simulink model "BswSigProc"  (R2022b) (continued)
== system BswSigProc == (continued)
  [SysServCarCfgSts_SysServDUIncarMotorSts_Tag] -> BswSigProc_Runnable_sys/93(SysServCarCfgSts_SysServDUIncarMotorSts_read)
  [SysServCarCfgSts_SysServDUIncarMotorSts_Tag] -> BswSigProc_SignalDeal_sys/48(SysServCarCfgSts_SysServDUIncarMotorSts_read)
  SysServCarCfgSts_SysServDUFRVentSts/1 -> [SysServCarCfgSts_SysServDUFRVentSts_Tag]
  [SysServCarCfgSts_SysServDUFRVentSts_Tag] -> BswSigProc_Runnable_sys/92(SysServCarCfgSts_SysServDUFRVentSts_read)
  [SysServCarCfgSts_SysServDUFRVentSts_Tag] -> BswSigProc_SignalDeal_sys/47(SysServCarCfgSts_SysServDUFRVentSts_read)
  SysServCarCfgSts_SysServDUFRFootSts/1 -> [SysServCarCfgSts_SysServDUFRFootSts_Tag]
  [SysServCarCfgSts_SysServDUFRFootSts_Tag] -> BswSigProc_Runnable_sys/91(SysServCarCfgSts_SysServDUFRFootSts_read)
  [SysServCarCfgSts_SysServDUFRFootSts_Tag] -> BswSigProc_SignalDeal_sys/46(SysServCarCfgSts_SysServDUFRFootSts_read)
  SysServCarCfgSts_SysServDUFLVentSts/1 -> [SysServCarCfgSts_SysServDUFLVentSts_Tag]
  [SysServCarCfgSts_SysServDUFLVentSts_Tag] -> BswSigProc_Runnable_sys/90(SysServCarCfgSts_SysServDUFLVentSts_read)
  [SysServCarCfgSts_SysServDUFLVentSts_Tag] -> BswSigProc_SignalDeal_sys/45(SysServCarCfgSts_SysServDUFLVentSts_read)
  SysServCarCfgSts_SysServDUFLFootSts/1 -> [SysServCarCfgSts_SysServDUFLFootSts_Tag]
  [SysServCarCfgSts_SysServDUFLFootSts_Tag] -> BswSigProc_Runnable_sys/89(SysServCarCfgSts_SysServDUFLFootSts_read)
  [SysServCarCfgSts_SysServDUFLFootSts_Tag] -> BswSigProc_SignalDeal_sys/44(SysServCarCfgSts_SysServDUFLFootSts_read)
  SysServCarCfgSts_SysServDUDCTRSts/1 -> [SysServCarCfgSts_SysServDUDCTRSts_Tag]
  [SysServCarCfgSts_SysServDUDCTRSts_Tag] -> BswSigProc_Runnable_sys/88(SysServCarCfgSts_SysServDUDCTRSts_read)
  [SysServCarCfgSts_SysServDUDCTRSts_Tag] -> BswSigProc_SignalDeal_sys/43(SysServCarCfgSts_SysServDUDCTRSts_read)
  SysServCarCfgSts_SysServDUDCTLSts/1 -> [SysServCarCfgSts_SysServDUDCTLSts_Tag]
  [SysServCarCfgSts_SysServDUDCTLSts_Tag] -> BswSigProc_Runnable_sys/87(SysServCarCfgSts_SysServDUDCTLSts_read)
  [SysServCarCfgSts_SysServDUDCTLSts_Tag] -> BswSigProc_SignalDeal_sys/42(SysServCarCfgSts_SysServDUDCTLSts_read)
  SysServCarCfgSts_SysServDUDCSTLSts/1 -> [SysServCarCfgSts_SysServDUDCSTLSts_Tag]
  [SysServCarCfgSts_SysServDUDCSTLSts_Tag] -> BswSigProc_Runnable_sys/86(SysServCarCfgSts_SysServDUDCSTLSts_read)
  [SysServCarCfgSts_SysServDUDCSTLSts_Tag] -> BswSigProc_SignalDeal_sys/41(SysServCarCfgSts_SysServDUDCSTLSts_read)
  SysServCarCfgSts_SysServDUDCSModeSts/1 -> [SysServCarCfgSts_SysServDUDCSModeSts_Tag]
  [SysServCarCfgSts_SysServDUDCSModeSts_Tag] -> BswSigProc_Runnable_sys/85(SysServCarCfgSts_SysServDUDCSModeSts_read)
  [SysServCarCfgSts_SysServDUDCSModeSts_Tag] -> BswSigProc_SignalDeal_sys/40(SysServCarCfgSts_SysServDUDCSModeSts_read)
  SysServCarCfgSts_SysServDUDCRecSts/1 -> [SysServCarCfgSts_SysServDUDCRecSts_Tag]
  [SysServCarCfgSts_SysServDUDCRecSts_Tag] -> BswSigProc_Runnable_sys/84(SysServCarCfgSts_SysServDUDCRecSts_read)
  [SysServCarCfgSts_SysServDUDCRecSts_Tag] -> BswSigProc_SignalDeal_sys/39(SysServCarCfgSts_SysServDUDCRecSts_read)
  SysServCarCfgSts_SysServDUDCModeSts/1 -> [SysServCarCfgSts_SysServDUDCModeSts_Tag]
  [SysServCarCfgSts_SysServDUDCModeSts_Tag] -> BswSigProc_Runnable_sys/83(SysServCarCfgSts_SysServDUDCModeSts_read)
  [SysServCarCfgSts_SysServDUDCModeSts_Tag] -> BswSigProc_SignalDeal_sys/38(SysServCarCfgSts_SysServDUDCModeSts_read)
  SysServCarCfgSts_SysServDUDCFLMdeSts/1 -> [SysServCarCfgSts_SysServDUDCFLMdeSts_Tag]
  [SysServCarCfgSts_SysServDUDCFLMdeSts_Tag] -> BswSigProc_Runnable_sys/82(SysServCarCfgSts_SysServDUDCFLMdeSts_read)
  [SysServCarCfgSts_SysServDUDCFLMdeSts_Tag] -> BswSigProc_SignalDeal_sys/37(SysServCarCfgSts_SysServDUDCFLMdeSts_read)
  SysServCarCfgSts_SysServDUDCDefrostSts/1 -> [SysServCarCfgSts_SysServDUDCDefrostSts_Tag]
  [SysServCarCfgSts_SysServDUDCDefrostSts_Tag] -> BswSigProc_Runnable_sys/81(SysServCarCfgSts_SysServDUDCDefrostSts_read)
  [SysServCarCfgSts_SysServDUDCDefrostSts_Tag] -> BswSigProc_SignalDeal_sys/36(SysServCarCfgSts_SysServDUDCDefrostSts_read)
  SysServCarCfgSts_SysServDUBlowerPwmSts/1 -> [SysServCarCfgSts_SysServDUBlowerPwmSts_Tag]
  [SysServCarCfgSts_SysServDUBlowerPwmSts_Tag] -> BswSigProc_Runnable_sys/80(SysServCarCfgSts_SysServDUBlowerPwmSts_read)
  [SysServCarCfgSts_SysServDUBlowerPwmSts_Tag] -> BswSigProc_SignalDeal_sys/35(SysServCarCfgSts_SysServDUBlowerPwmSts_read)
  SysServCarCfgSts_SysServDUBlowerPwmSignSts/1 -> [SysServCarCfgSts_SysServDUBlowerPwmSignSts_Tag]
  [SysServCarCfgSts_SysServDUBlowerPwmSignSts_Tag] -> BswSigProc_Runnable_sys/78(SysServCarCfgSts_SysServDUBlowerPwmSignSts_read)
  [SysServCarCfgSts_SysServDUBlowerPwmSignSts_Tag] -> BswSigProc_SignalDeal_sys/34(SysServCarCfgSts_SysServDUBlowerPwmSignSts_read)
  SysServCarCfgSts_SysServDUAirVentSnrSts/1 -> [SysServCarCfgSts_SysServDUAirVentSnrSts_Tag]
  [SysServCarCfgSts_SysServDUAirVentSnrSts_Tag] -> BswSigProc_Runnable_sys/77(SysServCarCfgSts_SysServDUAirVentSnrSts_read)
  [SysServCarCfgSts_SysServDUAirVentSnrSts_Tag] -> BswSigProc_SignalDeal_sys/33(SysServCarCfgSts_SysServDUAirVentSnrSts_read)
  SysServCarCfgSts_SysServCCPLin3VGASts/1 -> [SysServCarCfgSts_SysServCCPLin3VGASts_Tag]
  [SysServCarCfgSts_SysServCCPLin3VGASts_Tag] -> BswSigProc_Runnable_sys/76(SysServCarCfgSts_SysServCCPLin3VGASts_read)
  [SysServCarCfgSts_SysServCCPLin3VGASts_Tag] -> BswSigProc_SignalDeal_sys/32(SysServCarCfgSts_SysServCCPLin3VGASts_read)
  SysServCarCfgSts_SysServCCPLin2PMSISts/1 -> [SysServCarCfgSts_SysServCCPLin2PMSISts_Tag]
  [SysServCarCfgSts_SysServCCPLin2PMSISts_Tag] -> BswSigProc_Runnable_sys/75(SysServCarCfgSts_SysServCCPLin2PMSISts_read)
  [SysServCarCfgSts_SysServCCPLin2PMSISts_Tag] -> BswSigProc_SignalDeal_sys/31(SysServCarCfgSts_SysServCCPLin2PMSISts_read)
  SysServCarCfgSts_SysServCCPLin2CDSSts/1 -> [SysServCarCfgSts_SysServCCPLin2CDSSts_Tag]
  [SysServCarCfgSts_SysServCCPLin2CDSSts_Tag] -> BswSigProc_Runnable_sys/74(SysServCarCfgSts_SysServCCPLin2CDSSts_read)
  [SysServCarCfgSts_SysServCCPLin2CDSSts_Tag] -> BswSigProc_SignalDeal_sys/30(SysServCarCfgSts_SysServCCPLin2CDSSts_read)
  SysServCarCfgSts_SysServCCPLin2AIUSts/1 -> [SysServCarCfgSts_SysServCCPLin2AIUSts_Tag]
  [SysServCarCfgSts_SysServCCPLin2AIUSts_Tag] -> BswSigProc_Runnable_sys/72(SysServCarCfgSts_SysServCCPLin2AIUSts_read)
  [SysServCarCfgSts_SysServCCPLin2AIUSts_Tag] -> BswSigProc_SignalDeal_sys/29(SysServCarCfgSts_SysServCCPLin2AIUSts_read)
  SysServCarCfgSts_SysServCCPLin2AFUSts/1 -> [SysServCarCfgSts_SysServCCPLin2AFUSts_Tag]
  [SysServCarCfgSts_SysServCCPLin2AFUSts_Tag] -> BswSigProc_Runnable_sys/71(SysServCarCfgSts_SysServCCPLin2AFUSts_read)
  [SysServCarCfgSts_SysServCCPLin2AFUSts_Tag] -> BswSigProc_SignalDeal_sys/28(SysServCarCfgSts_SysServCCPLin2AFUSts_read)
  SysServCarCfgSts_SysServCCPHEXSensorSts/1 -> [SysServCarCfgSts_SysServCCPHEXSensorSts_Tag]
  [SysServCarCfgSts_SysServCCPHEXSensorSts_Tag] -> BswSigProc_Runnable_sys/70(SysServCarCfgSts_SysServCCPHEXSensorSts_read)
  [SysServCarCfgSts_SysServCCPHEXSensorSts_Tag] -> BswSigProc_SignalDeal_sys/27(SysServCarCfgSts_SysServCCPHEXSensorSts_read)
  SysServCarCfgSts_SysServCCPBGMNum/1 -> [SysServCarCfgSts_SysServCCPBGMNum_Tag]
  [SysServCarCfgSts_SysServCCPBGMNum_Tag] -> BswSigProc_Runnable_sys/69(SysServCarCfgSts_SysServCCPBGMNum_read)
  [SysServCarCfgSts_SysServCCPBGMNum_Tag] -> BswSigProc_SignalDeal_sys/99(SysServCarCfgSts_SysServCCPBGMNum_read)
  SysServCarCfgSts_SysServCCPAQSSts/1 -> [SysServCarCfgSts_SysServCCPAQSSts_Tag]
  [SysServCarCfgSts_SysServCCPAQSSts_Tag] -> BswSigProc_Runnable_sys/68(SysServCarCfgSts_SysServCCPAQSSts_read)
  [SysServCarCfgSts_SysServCCPAQSSts_Tag] -> BswSigProc_SignalDeal_sys/26(SysServCarCfgSts_SysServCCPAQSSts_read)
  SysServCarCfgSts_SysServCarID/1 -> [SysServCarCfgSts_SysServCarID_Tag]
  [SysServCarCfgSts_SysServCarID_Tag] -> BswSigProc_Runnable_sys/67(SysServCarCfgSts_SysServCarID_read)
  [SysServCarCfgSts_SysServCarID_Tag] -> BswSigProc_SignalDeal_sys/25(SysServCarCfgSts_SysServCarID_read)
  RlyCrashForClimaReq_Ngcc_RlyCrashForClimaReq/1 -> [RlyCrashForClimaReq_Ngcc_RlyCrashForClimaReq_Tag]
  [RlyCrashForClimaReq_Ngcc_RlyCrashForClimaReq_Tag] -> BswSigProc_Runnable_sys/66(RlyCrashForClimaReq_Ngcc_RlyCrashForClimaReq_read)
  [RlyCrashForClimaReq_Ngcc_RlyCrashForClimaReq_Tag] -> BswSigProc_SignalDeal_sys/24(RlyCrashForClimaReq_Ngcc_RlyCrashForClimaReq_read)
  RlyCmftForClimaReq_Ngcc_RlyCmftForClimaReq/1 -> [RlyCmftForClimaReq_Ngcc_RlyCmftForClimaReq_Tag]
  [RlyCmftForClimaReq_Ngcc_RlyCmftForClimaReq_Tag] -> BswSigProc_Runnable_sys/65(RlyCmftForClimaReq_Ngcc_RlyCmftForClimaReq_read)
  [RlyCmftForClimaReq_Ngcc_RlyCmftForClimaReq_Tag] -> BswSigProc_SignalDeal_sys/23(RlyCmftForClimaReq_Ngcc_RlyCmftForClimaReq_read)
  IntCo2RunSts_IntCo2RunSts_IsUpdated/1 -> [IntCo2RunSts_IntCo2RunSts_IsUpdated_Tag]
  [IntCo2RunSts_IntCo2RunSts_IsUpdated_Tag] -> BswSigProc_Runnable_sys/64(IntCo2RunSts_IntCo2RunSts_IsUpdated_read)
  [IntCo2RunSts_IntCo2RunSts_IsUpdated_Tag] -> BswSigProc_SignalDeal_sys/81(IntCo2RunSts_IntCo2RunSts_IsUpdated_read)
  IntCo2RunSts_IntCo2RunSts/1 -> [IntCo2RunSts_IntCo2RunSts_Tag]
  [IntCo2RunSts_IntCo2RunSts_Tag] -> BswSigProc_Runnable_sys/63(IntCo2RunSts_IntCo2RunSts_read)
  [IntCo2RunSts_IntCo2RunSts_Tag] -> BswSigProc_SignalDeal_sys/80(IntCo2RunSts_IntCo2RunSts_read)
  IntCo2Conc_IntCo2Conc_IsUpdated/1 -> [IntCo2Conc_IntCo2Conc_IsUpdated_Tag]
  [IntCo2Conc_IntCo2Conc_IsUpdated_Tag] -> BswSigProc_Runnable_sys/62(IntCo2Conc_IntCo2Conc_IsUpdated_read)
  [IntCo2Conc_IntCo2Conc_IsUpdated_Tag] -> BswSigProc_SignalDeal_sys/79(IntCo2Conc_IntCo2Conc_IsUpdated_read)
  IntCo2Conc_IntCo2Conc/1 -> [IntCo2Conc_IntCo2Conc_Tag]
  [IntCo2Conc_IntCo2Conc_Tag] -> BswSigProc_Runnable_sys/61(IntCo2Conc_IntCo2Conc_read)
  [IntCo2Conc_IntCo2Conc_Tag] -> BswSigProc_SignalDeal_sys/78(IntCo2Conc_IntCo2Conc_read)
  HvacOsaAndRecActrQfForHp_HvacOsaAndRecActrQfForHp/1 -> [HvacOsaAndRecActrQfForHp_HvacOsaAndRecActrQfForHp_Tag]
  [HvacOsaAndRecActrQfForHp_HvacOsaAndRecActrQfForHp_Tag] -> BswSigProc_Runnable_sys/59(HvacOsaAndRecActrQfForHp_HvacOsaAndRecActrQfForHp_read)
  [HvacOsaAndRecActrQfForHp_HvacOsaAndRecActrQfForHp_Tag] -> BswSigProc_SignalDeal_sys/21(HvacOsaAndRecActrQfForHp_HvacOsaAndRecActrQfForHp_read)
  HvacFanSts3HvacFanSpdFd_HvacFanSts3HvacFanSpdFd_IsUpdated/1 -> [HvacFanSts3HvacFanSpdFd_HvacFanSts3HvacFanSpdFd_IsUpdated_Tag]
  [HvacFanSts3HvacFanSpdFd_HvacFanSts3HvacFanSpdFd_IsUpdated_Tag] -> BswSigProc_Runnable_sys/58(HvacFanSts3HvacFanSpdFd_HvacFanSts3HvacFanSpdFd_IsUpdated_read)
  [HvacFanSts3HvacFanSpdFd_HvacFanSts3HvacFanSpdFd_IsUpdated_Tag] -> BswSigProc_SignalDeal_sys/77(HvacFanSts3HvacFanSpdFd_HvacFanSts3HvacFanSpdFd_IsUpdated_read)
  HvacFanSts3HvacFanSpdFd_HvacFanSts3HvacFanSpdFd/1 -> [HvacFanSts3HvacFanSpdFd_HvacFanSts3HvacFanSpdFd_Tag]
  [HvacFanSts3HvacFanSpdFd_HvacFanSts3HvacFanSpdFd_Tag] -> BswSigProc_Runnable_sys/57(HvacFanSts3HvacFanSpdFd_HvacFanSts3HvacFanSpdFd_read)
  [HvacFanSts3HvacFanSpdFd_HvacFanSts3HvacFanSpdFd_Tag] -> BswSigProc_SignalDeal_sys/76(HvacFanSts3HvacFanSpdFd_HvacFanSts3HvacFanSpdFd_read)
  HvacFanSts3HvacFanBattU_HvacFanSts3HvacFanBattU_IsUpdated/1 -> [HvacFanSts3HvacFanBattU_HvacFanSts3HvacFanBattU_IsUpdated_Tag]
  [HvacFanSts3HvacFanBattU_HvacFanSts3HvacFanBattU_IsUpdated_Tag] -> BswSigProc_Runnable_sys/56(HvacFanSts3HvacFanBattU_HvacFanSts3HvacFanBattU_IsUpdated_read)
  [HvacFanSts3HvacFanBattU_HvacFanSts3HvacFanBattU_IsUpdated_Tag] -> BswSigProc_SignalDeal_sys/75(HvacFanSts3HvacFanBattU_HvacFanSts3HvacFanBattU_IsUpdated_read)
  HvacFanSts3HvacFanBattU_HvacFanSts3HvacFanBattU/1 -> [HvacFanSts3HvacFanBattU_HvacFanSts3HvacFanBattU_Tag]
  [HvacFanSts3HvacFanBattU_HvacFanSts3HvacFanBattU_Tag] -> BswSigProc_Runnable_sys/55(HvacFanSts3HvacFanBattU_HvacFanSts3HvacFanBattU_read)
  [HvacFanSts3HvacFanBattU_HvacFanSts3HvacFanBattU_Tag] -> BswSigProc_SignalDeal_sys/74(HvacFanSts3HvacFanBattU_HvacFanSts3HvacFanBattU_read)
  HvacFanSts3HvacFanBattI_HvacFanSts3HvacFanBattI_IsUpdated/1 -> [HvacFanSts3HvacFanBattI_HvacFanSts3HvacFanBattI_IsUpdated_Tag]
  [HvacFanSts3HvacFanBattI_HvacFanSts3HvacFanBattI_IsUpdated_Tag] -> BswSigProc_Runnable_sys/54(HvacFanSts3HvacFanBattI_HvacFanSts3HvacFanBattI_IsUpdated_read)
  [HvacFanSts3HvacFanBattI_HvacFanSts3HvacFanBattI_IsUpdated_Tag] -> BswSigProc_SignalDeal_sys/73(HvacFanSts3HvacFanBattI_HvacFanSts3HvacFanBattI_IsUpdated_read)
  HvacFanSts3HvacFanBattI_HvacFanSts3HvacFanBattI/1 -> [HvacFanSts3HvacFanBattI_HvacFanSts3HvacFanBattI_Tag]
  [HvacFanSts3HvacFanBattI_HvacFanSts3HvacFanBattI_Tag] -> BswSigProc_Runnable_sys/53(HvacFanSts3HvacFanBattI_HvacFanSts3HvacFanBattI_read)
  [HvacFanSts3HvacFanBattI_HvacFanSts3HvacFanBattI_Tag] -> BswSigProc_SignalDeal_sys/72(HvacFanSts3HvacFanBattI_HvacFanSts3HvacFanBattI_read)
  HvacFanSts2HvacFanBattU2_HvacFanSts2HvacFanBattU2_IsUpdated/1 -> [HvacFanSts2HvacFanBattU2_HvacFanSts2HvacFanBattU2_IsUpdated_Tag]
  [HvacFanSts2HvacFanBattU2_HvacFanSts2HvacFanBattU2_IsUpdated_Tag] -> BswSigProc_Runnable_sys/52(HvacFanSts2HvacFanBattU2_HvacFanSts2HvacFanBattU2_IsUpdated_read)
  [HvacFanSts2HvacFanBattU2_HvacFanSts2HvacFanBattU2_IsUpdated_Tag] -> BswSigProc_SignalDeal_sys/71(HvacFanSts2HvacFanBattU2_HvacFanSts2HvacFanBattU2_IsUpdated_read)
  HvacFanSts2HvacFanBattU2_HvacFanSts2HvacFanBattU2/1 -> [HvacFanSts2HvacFanBattU2_HvacFanSts2HvacFanBattU2_Tag]
  [HvacFanSts2HvacFanBattU2_HvacFanSts2HvacFanBattU2_Tag] -> BswSigProc_Runnable_sys/51(HvacFanSts2HvacFanBattU2_HvacFanSts2HvacFanBattU2_read)
  [HvacFanSts2HvacFanBattU2_HvacFanSts2HvacFanBattU2_Tag] -> BswSigProc_SignalDeal_sys/70(HvacFanSts2HvacFanBattU2_HvacFanSts2HvacFanBattU2_read)
  HvacFanSts2HvacFanBattI2_HvacFanSts2HvacFanBattI2_IsUpdated/1 -> [HvacFanSts2HvacFanBattI2_HvacFanSts2HvacFanBattI2_IsUpdated_Tag]
  [HvacFanSts2HvacFanBattI2_HvacFanSts2HvacFanBattI2_IsUpdated_Tag] -> BswSigProc_Runnable_sys/50(HvacFanSts2HvacFanBattI2_HvacFanSts2HvacFanBattI2_IsUpdated_read)
  [HvacFanSts2HvacFanBattI2_HvacFanSts2HvacFanBattI2_IsUpdated_Tag] -> BswSigProc_SignalDeal_sys/69(HvacFanSts2HvacFanBattI2_HvacFanSts2HvacFanBattI2_IsUpdated_read)
  HvacFanSts2HvacFanBattI2_HvacFanSts2HvacFanBattI2/1 -> [HvacFanSts2HvacFanBattI2_HvacFanSts2HvacFanBattI2_Tag]
  [HvacFanSts2HvacFanBattI2_HvacFanSts2HvacFanBattI2_Tag] -> BswSigProc_Runnable_sys/49(HvacFanSts2HvacFanBattI2_HvacFanSts2HvacFanBattI2_read)
  [HvacFanSts2HvacFanBattI2_HvacFanSts2HvacFanBattI2_Tag] -> BswSigProc_SignalDeal_sys/68(HvacFanSts2HvacFanBattI2_HvacFanSts2HvacFanBattI2_read)
  HmiHvacFanLvlFrnt_HmiHvacFanLvlFrnt/1 -> [HmiHvacFanLvlFrnt_HmiHvacFanLvlFrnt_Tag]
  [HmiHvacFanLvlFrnt_HmiHvacFanLvlFrnt_Tag] -> BswSigProc_Runnable_sys/48(HmiHvacFanLvlFrnt_HmiHvacFanLvlFrnt_read)
  [HmiHvacFanLvlFrnt_HmiHvacFanLvlFrnt_Tag] -> BswSigProc_SignalDeal_sys/92(HmiHvacFanLvlFrnt_HmiHvacFanLvlFrnt_read)
  FrntHvacBlowerSts_FrntHvacBlowerSts/1 -> [FrntHvacBlowerSts_FrntHvacBlowerSts_Tag]
  [FrntHvacBlowerSts_FrntHvacBlowerSts_Tag] -> BswSigProc_Runnable_sys/47(FrntHvacBlowerSts_FrntHvacBlowerSts_read)
  [FrntHvacBlowerSts_FrntHvacBlowerSts_Tag] -> BswSigProc_SignalDeal_sys/20(FrntHvacBlowerSts_FrntHvacBlowerSts_read)
  CmptmtTRearRaw_CmptmtTRearRaw/1 -> [CmptmtTRearRaw_CmptmtTRearRaw_Tag]
  [CmptmtTRearRaw_CmptmtTRearRaw_Tag] -> BswSigProc_Runnable_sys/45(CmptmtTRearRaw_CmptmtTRearRaw_read)
  [CmptmtTRearRaw_CmptmtTRearRaw_Tag] -> BswSigProc_SignalDeal_sys/91(CmptmtTRearRaw_CmptmtTRearRaw_read)
  CarTiGlb_CarTiGlb_IsUpdated/1 -> [CarTiGlb_CarTiGlb_IsUpdated_Tag]
  [CarTiGlb_CarTiGlb_IsUpdated_Tag] -> BswSigProc_Runnable_sys/44(CarTiGlb_CarTiGlb_IsUpdated_read)
  [CarTiGlb_CarTiGlb_IsUpdated_Tag] -> BswSigProc_SignalDeal_sys/88(CarTiGlb_CarTiGlb_IsUpdated_read)
  CarTiGlb_CarTiGlb/1 -> [CarTiGlb_CarTiGlb_Tag]
  [CarTiGlb_CarTiGlb_Tag] -> BswSigProc_Runnable_sys/43(CarTiGlb_CarTiGlb_read)
  [CarTiGlb_CarTiGlb_Tag] -> BswSigProc_SignalDeal_sys/87(CarTiGlb_CarTiGlb_read)
  BkpOfDstTrvld_BkpOfDstTrvld_IsUpdated/1 -> [BkpOfDstTrvld_BkpOfDstTrvld_IsUpdated_Tag]
  [BkpOfDstTrvld_BkpOfDstTrvld_IsUpdated_Tag] -> BswSigProc_Runnable_sys/42(BkpOfDstTrvld_BkpOfDstTrvld_IsUpdated_read)
  [BkpOfDstTrvld_BkpOfDstTrvld_IsUpdated_Tag] -> BswSigProc_SignalDeal_sys/86(BkpOfDstTrvld_BkpOfDstTrvld_IsUpdated_read)
  BkpOfDstTrvld_BkpOfDstTrvld/1 -> [BkpOfDstTrvld_BkpOfDstTrvld_Tag]
  [BkpOfDstTrvld_BkpOfDstTrvld_Tag] -> BswSigProc_Runnable_sys/41(BkpOfDstTrvld_BkpOfDstTrvld_read)
  [BkpOfDstTrvld_BkpOfDstTrvld_Tag] -> BswSigProc_SignalDeal_sys/85(BkpOfDstTrvld_BkpOfDstTrvld_read)
  BatteryVolValue_BatteryVolValue/1 -> [BatteryVolValue_BatteryVolValue_Tag]
  [BatteryVolValue_BatteryVolValue_Tag] -> BswSigProc_Runnable_sys/40(BatteryVolValue_BatteryVolValue_read)
  [BatteryVolValue_BatteryVolValue_Tag] -> BswSigProc_SignalDeal_sys/18(BatteryVolValue_BatteryVolValue_read)
  AmbTRaw_AmbTRaw/1 -> [AmbTRaw_AmbTRaw_Tag]
  [AmbTRaw_AmbTRaw_Tag] -> BswSigProc_Runnable_sys/39(AmbTRaw_AmbTRaw_read)
  [AmbTRaw_AmbTRaw_Tag] -> BswSigProc_SignalDeal_sys/84(AmbTRaw_AmbTRaw_read)
  AirFragCh5RunngSts_AirFragCh5RunngSts_IsUpdated/1 -> [AirFragCh5RunngSts_AirFragCh5RunngSts_IsUpdated_Tag]
  [AirFragCh5RunngSts_AirFragCh5RunngSts_IsUpdated_Tag] -> BswSigProc_Runnable_sys/38(AirFragCh5RunngSts_AirFragCh5RunngSts_IsUpdated_read)
  [AirFragCh5RunngSts_AirFragCh5RunngSts_IsUpdated_Tag] -> BswSigProc_SignalDeal_sys/67(AirFragCh5RunngSts_AirFragCh5RunngSts_IsUpdated_read)
  AirFragCh5RunngSts_AirFragCh5RunngSts/1 -> [AirFragCh5RunngSts_AirFragCh5RunngSts_Tag]
  [AirFragCh5RunngSts_AirFragCh5RunngSts_Tag] -> BswSigProc_Runnable_sys/37(AirFragCh5RunngSts_AirFragCh5RunngSts_read)
  [AirFragCh5RunngSts_AirFragCh5RunngSts_Tag] -> BswSigProc_SignalDeal_sys/66(AirFragCh5RunngSts_AirFragCh5RunngSts_read)
  AirFragCh4RunngSts_AirFragCh4RunngSts_IsUpdated/1 -> [AirFragCh4RunngSts_AirFragCh4RunngSts_IsUpdated_Tag]
  [AirFragCh4RunngSts_AirFragCh4RunngSts_IsUpdated_Tag] -> BswSigProc_Runnable_sys/36(AirFragCh4RunngSts_AirFragCh4RunngSts_IsUpdated_read)
  [AirFragCh4RunngSts_AirFragCh4RunngSts_IsUpdated_Tag] -> BswSigProc_SignalDeal_sys/65(AirFragCh4RunngSts_AirFragCh4RunngSts_IsUpdated_read)
  AirFragCh4RunngSts_AirFragCh4RunngSts/1 -> [AirFragCh4RunngSts_AirFragCh4RunngSts_Tag]
  [AirFragCh4RunngSts_AirFragCh4RunngSts_Tag] -> BswSigProc_Runnable_sys/35(AirFragCh4RunngSts_AirFragCh4RunngSts_read)
  [AirFragCh4RunngSts_AirFragCh4RunngSts_Tag] -> BswSigProc_SignalDeal_sys/64(AirFragCh4RunngSts_AirFragCh4RunngSts_read)
  AirFragCh3RunngSts_AirFragCh3RunngSts_IsUpdated/1 -> [AirFragCh3RunngSts_AirFragCh3RunngSts_IsUpdated_Tag]
  [AirFragCh3RunngSts_AirFragCh3RunngSts_IsUpdated_Tag] -> BswSigProc_Runnable_sys/34(AirFragCh3RunngSts_AirFragCh3RunngSts_IsUpdated_read)
  [AirFragCh3RunngSts_AirFragCh3RunngSts_IsUpdated_Tag] -> BswSigProc_SignalDeal_sys/63(AirFragCh3RunngSts_AirFragCh3RunngSts_IsUpdated_read)
  AirFragCh3RunngSts_AirFragCh3RunngSts/1 -> [AirFragCh3RunngSts_AirFragCh3RunngSts_Tag]
  [AirFragCh3RunngSts_AirFragCh3RunngSts_Tag] -> BswSigProc_Runnable_sys/33(AirFragCh3RunngSts_AirFragCh3RunngSts_read)
  [AirFragCh3RunngSts_AirFragCh3RunngSts_Tag] -> BswSigProc_SignalDeal_sys/62(AirFragCh3RunngSts_AirFragCh3RunngSts_read)
  AirFragCh2RunngSts_AirFragCh2RunngSts_IsUpdated/1 -> [AirFragCh2RunngSts_AirFragCh2RunngSts_IsUpdated_Tag]
  [AirFragCh2RunngSts_AirFragCh2RunngSts_IsUpdated_Tag] -> BswSigProc_Runnable_sys/32(AirFragCh2RunngSts_AirFragCh2RunngSts_IsUpdated_read)
  [AirFragCh2RunngSts_AirFragCh2RunngSts_IsUpdated_Tag] -> BswSigProc_SignalDeal_sys/61(AirFragCh2RunngSts_AirFragCh2RunngSts_IsUpdated_read)
  AirFragCh2RunngSts_AirFragCh2RunngSts/1 -> [AirFragCh2RunngSts_AirFragCh2RunngSts_Tag]
  [AirFragCh2RunngSts_AirFragCh2RunngSts_Tag] -> BswSigProc_Runnable_sys/31(AirFragCh2RunngSts_AirFragCh2RunngSts_read)
  [AirFragCh2RunngSts_AirFragCh2RunngSts_Tag] -> BswSigProc_SignalDeal_sys/60(AirFragCh2RunngSts_AirFragCh2RunngSts_read)
  AirFragCh1RunngSts_AirFragCh1RunngSts_IsUpdated/1 -> [AirFragCh1RunngSts_AirFragCh1RunngSts_IsUpdated_Tag]
  [AirFragCh1RunngSts_AirFragCh1RunngSts_IsUpdated_Tag] -> BswSigProc_Runnable_sys/30(AirFragCh1RunngSts_AirFragCh1RunngSts_IsUpdated_read)
  [AirFragCh1RunngSts_AirFragCh1RunngSts_IsUpdated_Tag] -> BswSigProc_SignalDeal_sys/83(AirFragCh1RunngSts_AirFragCh1RunngSts_IsUpdated_read)
  AirFragCh1RunngSts_AirFragCh1RunngSts/1 -> [AirFragCh1RunngSts_AirFragCh1RunngSts_Tag]
  [AirFragCh1RunngSts_AirFragCh1RunngSts_Tag] -> BswSigProc_Runnable_sys/29(AirFragCh1RunngSts_AirFragCh1RunngSts_read)
  [AirFragCh1RunngSts_AirFragCh1RunngSts_Tag] -> BswSigProc_SignalDeal_sys/82(AirFragCh1RunngSts_AirFragCh1RunngSts_read)
  AirFlapPosnFrntActForRowSecForActrTRi_AirFlapPosnFrntActForRowSecForActrTRi/1 -> [AirFlapPosnFrntActForRowSecForActrTRi_AirFlapP_9fab42d88a26ba35]
  [AirFlapPosnFrntActForRowSecForActrTRi_AirFlapP_9fab42d88a26ba35] -> BswSigProc_Runnable_sys/28(AirFlapPosnFrntActForRowSecForActrTRi_AirFlapPosnFrntActForRowSecForActrTRi_read)
  [AirFlapPosnFrntActForRowSecForActrTRi_AirFlapP_9fab42d88a26ba35] -> BswSigProc_SignalDeal_sys/17(AirFlapPosnFrntActForRowSecForActrTRi_AirFlapPosnFrntActForRowSecForActrTRi_read)
  AirFlapPosnFrntActForRowSecForActrTLe_AirFlapPosnFrntActForRowSecForActrTLe/1 -> [AirFlapPosnFrntActForRowSecForActrTLe_AirFlapP_d65546ff01a636a1]
  [AirFlapPosnFrntActForRowSecForActrTLe_AirFlapP_d65546ff01a636a1] -> BswSigProc_Runnable_sys/27(AirFlapPosnFrntActForRowSecForActrTLe_AirFlapPosnFrntActForRowSecForActrTLe_read)
  [AirFlapPosnFrntActForRowSecForActrTLe_AirFlapP_d65546ff01a636a1] -> BswSigProc_SignalDeal_sys/16(AirFlapPosnFrntActForRowSecForActrTLe_AirFlapPosnFrntActForRowSecForActrTLe_read)
  AirFlapPosnFrntActForRowFirstForRecirc_AirFlapPosnFrntActForRowFirstForRecirc/1 -> [AirFlapPosnFrntActForRowFirstForRecirc_AirFlap_171d170c661908da]
  [AirFlapPosnFrntActForRowFirstForRecirc_AirFlap_171d170c661908da] -> BswSigProc_Runnable_sys/26(AirFlapPosnFrntActForRowFirstForRecirc_AirFlapPosnFrntActForRowFirstForRecirc_read)
  [AirFlapPosnFrntActForRowFirstForRecirc_AirFlap_171d170c661908da] -> BswSigProc_SignalDeal_sys/15(AirFlapPosnFrntActForRowFirstForRecirc_AirFlapPosnFrntActForRowFirstForRecirc_read)
  AirFlapPosnFrntActForRowFirstForOsa_AirFlapPosnFrntActForRowFirstForOsa/1 -> [AirFlapPosnFrntActForRowFirstForOsa_AirFlapPos_c9756757d150e2e6]
  [AirFlapPosnFrntActForRowFirstForOsa_AirFlapPos_c9756757d150e2e6] -> BswSigProc_Runnable_sys/25(AirFlapPosnFrntActForRowFirstForOsa_AirFlapPosnFrntActForRowFirstForOsa_read)
  [AirFlapPosnFrntActForRowFirstForOsa_AirFlapPos_c9756757d150e2e6] -> BswSigProc_SignalDeal_sys/14(AirFlapPosnFrntActForRowFirstForOsa_AirFlapPosnFrntActForRowFirstForOsa_read)
  AirFlapPosnFrntActForRowFirstForActrVentSideRi_AirFlapPosnFrntActForRowFirstForActrVentSideRi/1 -> [AirFlapPosnFrntActForRowFirstForActrVentSideRi_6aad2948aa0ad165]
  [AirFlapPosnFrntActForRowFirstForActrVentSideRi_6aad2948aa0ad165] -> BswSigProc_Runnable_sys/24(AirFlapPosnFrntActForRowFirstForActrVentSideRi_AirFlapPosnFrntActForRowFirstForActrVentSideRi_read)
  [AirFlapPosnFrntActForRowFirstForActrVentSideRi_6aad2948aa0ad165] -> BswSigProc_SignalDeal_sys/13(AirFlapPosnFrntActForRowFirstForActrVentSideRi_AirFlapPosnFrntActForRowFirstForActrVentSideRi_read)
  AirFlapPosnFrntActForRowFirstForActrVentSideLe_AirFlapPosnFrntActForRowFirstForActrVentSideLe/1 -> [AirFlapPosnFrntActForRowFirstForActrVentSideLe_15947c05f7951f1c]
  [AirFlapPosnFrntActForRowFirstForActrVentSideLe_15947c05f7951f1c] -> BswSigProc_Runnable_sys/23(AirFlapPosnFrntActForRowFirstForActrVentSideLe_AirFlapPosnFrntActForRowFirstForActrVentSideLe_read)
  [AirFlapPosnFrntActForRowFirstForActrVentSideLe_15947c05f7951f1c] -> BswSigProc_SignalDeal_sys/12(AirFlapPosnFrntActForRowFirstForActrVentSideLe_AirFlapPosnFrntActForRowFirstForActrVentSideLe_read)
  AirFlapPosnFrntActForRowFirstForActrVentRi_AirFlapPosnFrntActForRowFirstForActrVentRi/1 -> [AirFlapPosnFrntActForRowFirstForActrVentRi_Air_b5a3291dda15e940]
  [AirFlapPosnFrntActForRowFirstForActrVentRi_Air_b5a3291dda15e940] -> BswSigProc_Runnable_sys/22(AirFlapPosnFrntActForRowFirstForActrVentRi_AirFlapPosnFrntActForRowFirstForActrVentRi_read)
  [AirFlapPosnFrntActForRowFirstForActrVentRi_Air_b5a3291dda15e940] -> BswSigProc_SignalDeal_sys/11(AirFlapPosnFrntActForRowFirstForActrVentRi_AirFlapPosnFrntActForRowFirstForActrVentRi_read)
  AirFlapPosnFrntActForRowFirstForActrVentLe_AirFlapPosnFrntActForRowFirstForActrVentLe/1 -> [AirFlapPosnFrntActForRowFirstForActrVentLe_Air_c3694509e6d801f1]
  [AirFlapPosnFrntActForRowFirstForActrVentLe_Air_c3694509e6d801f1] -> BswSigProc_Runnable_sys/21(AirFlapPosnFrntActForRowFirstForActrVentLe_AirFlapPosnFrntActForRowFirstForActrVentLe_read)
  [AirFlapPosnFrntActForRowFirstForActrVentLe_Air_c3694509e6d801f1] -> BswSigProc_SignalDeal_sys/10(AirFlapPosnFrntActForRowFirstForActrVentLe_AirFlapPosnFrntActForRowFirstForActrVentLe_read)
  AirFlapPosnFrntActForRowFirstForActrTRi_AirFlapPosnFrntActForRowFirstForActrTRi/1 -> [AirFlapPosnFrntActForRowFirstForActrTRi_AirFla_b27cb14619f1f624]
  [AirFlapPosnFrntActForRowFirstForActrTRi_AirFla_b27cb14619f1f624] -> BswSigProc_Runnable_sys/20(AirFlapPosnFrntActForRowFirstForActrTRi_AirFlapPosnFrntActForRowFirstForActrTRi_read)
  [AirFlapPosnFrntActForRowFirstForActrTRi_AirFla_b27cb14619f1f624] -> BswSigProc_SignalDeal_sys/9(AirFlapPosnFrntActForRowFirstForActrTRi_AirFlapPosnFrntActForRowFirstForActrTRi_read)
  AirFlapPosnFrntActForRowFirstForActrTLe_AirFlapPosnFrntActForRowFirstForActrTLe/1 -> [AirFlapPosnFrntActForRowFirstForActrTLe_AirFla_7693c71f9709e146]
  [AirFlapPosnFrntActForRowFirstForActrTLe_AirFla_7693c71f9709e146] -> BswSigProc_Runnable_sys/19(AirFlapPosnFrntActForRowFirstForActrTLe_AirFlapPosnFrntActForRowFirstForActrTLe_read)
  [AirFlapPosnFrntActForRowFirstForActrTLe_AirFla_7693c71f9709e146] -> BswSigProc_SignalDeal_sys/8(AirFlapPosnFrntActForRowFirstForActrTLe_AirFlapPosnFrntActForRowFirstForActrTLe_read)
  AirFlapPosnFrntActForRowFirstForActrFlrRi_AirFlapPosnFrntActForRowFirstForActrFlrRi/1 -> [AirFlapPosnFrntActForRowFirstForActrFlrRi_AirF_adb4d68377445f03]
  [AirFlapPosnFrntActForRowFirstForActrFlrRi_AirF_adb4d68377445f03] -> BswSigProc_Runnable_sys/18(AirFlapPosnFrntActForRowFirstForActrFlrRi_AirFlapPosnFrntActForRowFirstForActrFlrRi_read)
  [AirFlapPosnFrntActForRowFirstForActrFlrRi_AirF_adb4d68377445f03] -> BswSigProc_SignalDeal_sys/7(AirFlapPosnFrntActForRowFirstForActrFlrRi_AirFlapPosnFrntActForRowFirstForActrFlrRi_read)
  AirFlapPosnFrntActForRowFirstForActrFlrLe_AirFlapPosnFrntActForRowFirstForActrFlrLe/1 -> [AirFlapPosnFrntActForRowFirstForActrFlrLe_AirF_9eebcd7ae28317ae]
  [AirFlapPosnFrntActForRowFirstForActrFlrLe_AirF_9eebcd7ae28317ae] -> BswSigProc_Runnable_sys/17(AirFlapPosnFrntActForRowFirstForActrFlrLe_AirFlapPosnFrntActForRowFirstForActrFlrLe_read)
  [AirFlapPosnFrntActForRowFirstForActrFlrLe_AirF_9eebcd7ae28317ae] -> BswSigProc_SignalDeal_sys/6(AirFlapPosnFrntActForRowFirstForActrFlrLe_AirFlapPosnFrntActForRowFirstForActrFlrLe_read)
  AirFlapPosnFrntActForRowFirstForActrDefrst_AirFlapPosnFrntActForRowFirstForActrDefrst/1 -> [AirFlapPosnFrntActForRowFirstForActrDefrst_Air_c6000bf2afa41b52]
  [AirFlapPosnFrntActForRowFirstForActrDefrst_Air_c6000bf2afa41b52] -> BswSigProc_Runnable_sys/16(AirFlapPosnFrntActForRowFirstForActrDefrst_AirFlapPosnFrntActForRowFirstForActrDefrst_read)
  [AirFlapPosnFrntActForRowFirstForActrDefrst_Air_c6000bf2afa41b52] -> BswSigProc_SignalDeal_sys/5(AirFlapPosnFrntActForRowFirstForActrDefrst_AirFlapPosnFrntActForRowFirstForActrDefrst_read)
  ActrPosnFrntActForRowSecModDistbnRi_ActrPosnFrntActForRowSecModDistbnRi/1 -> [ActrPosnFrntActForRowSecModDistbnRi_ActrPosnFr_81b854224ffc48df]
  [ActrPosnFrntActForRowSecModDistbnRi_ActrPosnFr_81b854224ffc48df] -> BswSigProc_Runnable_sys/15(ActrPosnFrntActForRowSecModDistbnRi_ActrPosnFrntActForRowSecModDistbnRi_read)
  [ActrPosnFrntActForRowSecModDistbnRi_ActrPosnFr_81b854224ffc48df] -> BswSigProc_SignalDeal_sys/4(ActrPosnFrntActForRowSecModDistbnRi_ActrPosnFrntActForRowSecModDistbnRi_read)
  ActrPosnFrntActForRowSecModDistbnLe_ActrPosnFrntActForRowSecModDistbnLe/1 -> [ActrPosnFrntActForRowSecModDistbnLe_ActrPosnFr_85d8778f0e81bbf3]
  [ActrPosnFrntActForRowSecModDistbnLe_ActrPosnFr_85d8778f0e81bbf3] -> BswSigProc_Runnable_sys/14(ActrPosnFrntActForRowSecModDistbnLe_ActrPosnFrntActForRowSecModDistbnLe_read)
  [ActrPosnFrntActForRowSecModDistbnLe_ActrPosnFr_85d8778f0e81bbf3] -> BswSigProc_SignalDeal_sys/3(ActrPosnFrntActForRowSecModDistbnLe_ActrPosnFrntActForRowSecModDistbnLe_read)
  ActrPosnFrntActForRowFirstModDistbn_ActrPosnFrntActForRowFirstModDistbn/1 -> [ActrPosnFrntActForRowFirstModDistbn_ActrPosnFr_ef360f37bfc032df]
  [ActrPosnFrntActForRowFirstModDistbn_ActrPosnFr_ef360f37bfc032df] -> BswSigProc_Runnable_sys/13(ActrPosnFrntActForRowFirstModDistbn_ActrPosnFrntActForRowFirstModDistbn_read)
  [ActrPosnFrntActForRowFirstModDistbn_ActrPosnFr_ef360f37bfc032df] -> BswSigProc_SignalDeal_sys/2(ActrPosnFrntActForRowFirstModDistbn_ActrPosnFrntActForRowFirstModDistbn_read)
  ActrPosnFrntActForOsaAndRecirc_ActrPosnFrntActForOsaAndRecirc/1 -> [ActrPosnFrntActForOsaAndRecirc_ActrPosnFrntAct_e7ddea020e0995f6]
  [ActrPosnFrntActForOsaAndRecirc_ActrPosnFrntAct_e7ddea020e0995f6] -> BswSigProc_Runnable_sys/12(ActrPosnFrntActForOsaAndRecirc_ActrPosnFrntActForOsaAndRecirc_read)
  [ActrPosnFrntActForOsaAndRecirc_ActrPosnFrntAct_e7ddea020e0995f6] -> BswSigProc_SignalDeal_sys/1(ActrPosnFrntActForOsaAndRecirc_ActrPosnFrntActForOsaAndRecirc_read)
  RlyCmdCmft_RlyCmdCmft/1 -> [RlyCmdCmft_RlyCmdCmft_Tag]
  [RlyCmdCmft_RlyCmdCmft_Tag] -> BswSigProc_Runnable_sys/11(RlyCmdCmft_RlyCmdCmft_read)
  [RlyCmdCmft_RlyCmdCmft_Tag] -> BswSigProc_SignalDeal_sys/98(RlyCmdCmft_RlyCmdCmft_read)
  PwrGernalOn2OffCnt_PwrMdGernalOn2OffCnt/1 -> [PwrGernalOn2OffCnt_PwrMdGernalOn2OffCnt_Tag]
  [PwrGernalOn2OffCnt_PwrMdGernalOn2OffCnt_Tag] -> BswSigProc_Runnable_sys/10(PwrGernalOn2OffCnt_PwrMdGernalOn2OffCnt_read)
  [PwrGernalOn2OffCnt_PwrMdGernalOn2OffCnt_Tag] -> BswSigProc_SignalDeal_sys/22(PwrGernalOn2OffCnt_PwrMdGernalOn2OffCnt_read)
  PrkgClimaActvMgrVFCWindows_PrkgClimaActvMgrVFCWindows/1 -> [PrkgClimaActvMgrVFCWindows_PrkgClimaActvMgrVFCWindows_Tag]
  [PrkgClimaActvMgrVFCWindows_PrkgClimaActvMgrVFCWindows_Tag] -> BswSigProc_Runnable_sys/9(PrkgClimaActvMgrVFCWindows_PrkgClimaActvMgrVFCWindows_read)
  [PrkgClimaActvMgrVFCWindows_PrkgClimaActvMgrVFCWindows_Tag] -> BswSigProc_SignalDeal_sys/97(PrkgClimaActvMgrVFCWindows_PrkgClimaActvMgrVFCWindows_read)
  PrkgClimaActvMgrVFCPostClimatisation_PrkgClimaActvMgrVFCPostClimatisation/1 -> [PrkgClimaActvMgrVFCPostClimatisation_PrkgClima_63912f1967780b64]
  [PrkgClimaActvMgrVFCPostClimatisation_PrkgClima_63912f1967780b64] -> BswSigProc_Runnable_sys/8(PrkgClimaActvMgrVFCPostClimatisation_PrkgClimaActvMgrVFCPostClimatisation_read)
  [PrkgClimaActvMgrVFCPostClimatisation_PrkgClima_63912f1967780b64] -> BswSigProc_SignalDeal_sys/96(PrkgClimaActvMgrVFCPostClimatisation_PrkgClimaActvMgrVFCPostClimatisation_read)
  PrkgClimaActvMgrVFCParkingDrivingClimatization_PrkgClimaActvMgrVFCParkingDrivingClimatization/1 -> [PrkgClimaActvMgrVFCParkingDrivingClimatization_3f3ca5cb54e1b4ce]
  [PrkgClimaActvMgrVFCParkingDrivingClimatization_3f3ca5cb54e1b4ce] -> BswSigProc_Runnable_sys/7(PrkgClimaActvMgrVFCParkingDrivingClimatization_PrkgClimaActvMgrVFCParkingDrivingClimatization_read)
  [PrkgClimaActvMgrVFCParkingDrivingClimatization_3f3ca5cb54e1b4ce] -> BswSigProc_SignalDeal_sys/95(PrkgClimaActvMgrVFCParkingDrivingClimatization_PrkgClimaActvMgrVFCParkingDrivingClimatization_read)
  PrkgClimaActvMgrVFCLocking_PrkgClimaActvMgrVFCLocking/1 -> [PrkgClimaActvMgrVFCLocking_PrkgClimaActvMgrVFCLocking_Tag]
  [PrkgClimaActvMgrVFCLocking_PrkgClimaActvMgrVFCLocking_Tag] -> BswSigProc_Runnable_sys/6(PrkgClimaActvMgrVFCLocking_PrkgClimaActvMgrVFCLocking_read)
  [PrkgClimaActvMgrVFCLocking_PrkgClimaActvMgrVFCLocking_Tag] -> BswSigProc_SignalDeal_sys/94(PrkgClimaActvMgrVFCLocking_PrkgClimaActvMgrVFCLocking_read)
  PrkgClimaActvMgrVFCGlobalShortDuration_PrkgClimaActvMgrVFCGlobalShortDuration/1 -> [PrkgClimaActvMgrVFCGlobalShortDuration_PrkgCli_a65a1557b23b126a]
  [PrkgClimaActvMgrVFCGlobalShortDuration_PrkgCli_a65a1557b23b126a] -> BswSigProc_Runnable_sys/5(PrkgClimaActvMgrVFCGlobalShortDuration_PrkgClimaActvMgrVFCGlobalShortDuration_read)
  [PrkgClimaActvMgrVFCGlobalShortDuration_PrkgCli_a65a1557b23b126a] -> BswSigProc_SignalDeal_sys/93(PrkgClimaActvMgrVFCGlobalShortDuration_PrkgClimaActvMgrVFCGlobalShortDuration_read)
  PrkgClimaActvMgrVFCBodyPreClimatisation_PrkgClimaActvMgrVFCBodyPreClimatisation/1 -> [PrkgClimaActvMgrVFCBodyPreClimatisation_PrkgCl_001834cbe5d5ad92]
  [PrkgClimaActvMgrVFCBodyPreClimatisation_PrkgCl_001834cbe5d5ad92] -> BswSigProc_Runnable_sys/4(PrkgClimaActvMgrVFCBodyPreClimatisation_PrkgClimaActvMgrVFCBodyPreClimatisation_read)
  [PrkgClimaActvMgrVFCBodyPreClimatisation_PrkgCl_001834cbe5d5ad92] -> BswSigProc_SignalDeal_sys/19(PrkgClimaActvMgrVFCBodyPreClimatisation_PrkgClimaActvMgrVFCBodyPreClimatisation_read)
  CmptmtTFrntRaw_CmptmtTFrntRaw/1 -> [CmptmtTFrntRaw_CmptmtTFrntRaw_Tag]
  [CmptmtTFrntRaw_CmptmtTFrntRaw_Tag] -> BswSigProc_Runnable_sys/3(CmptmtTFrntRaw_CmptmtTFrntRaw_read)
  [CmptmtTFrntRaw_CmptmtTFrntRaw_Tag] -> BswSigProc_SignalDeal_sys/59(CmptmtTFrntRaw_CmptmtTFrntRaw_read)
  ClimRlyCmd_ClimRlyCmd/1 -> [ClimRlyCmd_ClimRlyCmd_Tag]
  [ClimRlyCmd_ClimRlyCmd_Tag] -> BswSigProc_Runnable_sys/2(ClimRlyCmd_ClimRlyCmd_read)
  [ClimRlyCmd_ClimRlyCmd_Tag] -> BswSigProc_SignalDeal_sys/90(ClimRlyCmd_ClimRlyCmd_read)
  ClimaTiMgrVFCInfotainmentPush_ClimaTiMgrVFCInfotainmentPush/1 -> [ClimaTiMgrVFCInfotainmentPush_ClimaTiMgrVFCInfotainmentPush_Tag]
  [ClimaTiMgrVFCInfotainmentPush_ClimaTiMgrVFCInfotainmentPush_Tag] -> BswSigProc_Runnable_sys/1(ClimaTiMgrVFCInfotainmentPush_ClimaTiMgrVFCInfotainmentPush_read)
  [ClimaTiMgrVFCInfotainmentPush_ClimaTiMgrVFCInfotainmentPush_Tag] -> BswSigProc_SignalDeal_sys/89(ClimaTiMgrVFCInfotainmentPush_ClimaTiMgrVFCInfotainmentPush_read)
  BswSigProc_Runnable/1 -> BswSigProc_Runnable_sys/Trigger
  BswSigProc_SignalDeal/1 -> BswSigProc_SignalDeal_sys/Trigger
  Xcp_Event0/1 -> Xcp_Event0_sys/Trigger
  Xcp_Event1/1 -> Xcp_Event1_sys/Trigger
  Xcp_Event2/1 -> Xcp_Event2_sys/Trigger
  Xcp_Event3/1 -> Xcp_Event3_sys/Trigger
  Xcp_Event4/1 -> Xcp_Event4_sys/Trigger
  Xcp_Event5/1 -> Xcp_Event5_sys/Trigger
